simulink model "SdbTxSigProc"  (R2022b)
settings: Solver=FixedStepDiscrete  StartTime=0.0  StopTime=10.0  FixedStep=0.1  RelTol=1e-3
(part 3 of 4)

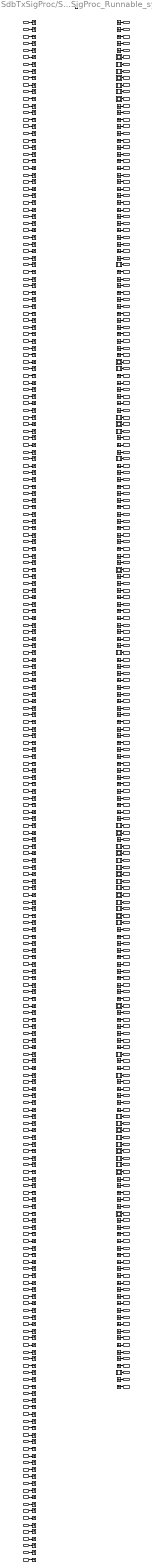
== system SdbTxSigProc/SdbTxSigProc_Runnable_sys ==
  AirTAftHvacDes_AirTAftHvacDes_read : Inport
  AirTFbFromClima_Ngcc_AirTFbFromClima_read : Inport  Port=2
  AmbTEstimd_Ngcc_AmbTEstimd_read : Inport  Port=3
  AmbTFildForClimaNgcc_AmbTFildForClima_read : Inport  Port=4
  AmbTFildForClimaQf_AmbTFildForClimaQf_read : Inport  Port=5
  CdsnProbltyToExt_CdsnProbltyToExt_read : Inport  Port=6
  ClimaActv_Ngcc_ClimaActv_read : Inport  Port=7
  ClimaActvAlrmSts_Ngcc_ClimaActvAlrmSts_read : Inport  Port=8
  ClimaCoolgPwrThermPred_ClimaCoolgPwrThermPred_read : Inport  Port=9
  ClimaDefrstMaxReq_ClimaDefrstMaxReq_read : Inport  Port=10
  ClimaOffReq_Ngcc_ClimaOffReq_read : Inport  Port=11
  ClimaPwrCns_Ngcc_ClimaPwrCns_read : Inport  Port=12
  ClimaPwrCnsPred_ClimaPwrCnsPred_read : Inport  Port=13
  ClimaPwrCritDes_Ngcc_ClimaPwrCritDes_read : Inport  Port=14
  ClimaPwrNormDes_Ngcc_ClimaPwrNormDes_read : Inport  Port=15
  ClimaStrtTi_ClimaStrtTi_read : Inport  Port=16
  ClimaSts_Ngcc_ClimaSts_read : Inport  Port=17
  ClimaTiOfEnd_Ngcc_ClimaTiOfEnd_read : Inport  Port=18
  ClimaTmrSts_ClimaTmrSts_read : Inport  Port=19
  ClimaWarn_ClimaWarn_read : Inport  Port=20
  ClngActv_ClngActv_read : Inport  Port=21
  CmptmtAirTEstimdAtRowFirstLoResl_Ngcc_CmptmtAirTEstimdAtRowFirstLoResl_read : Inport  Port=22
  CmptmtAirTEstimdExtd_Ngcc_CmptmtAirTEstimdExtd_read : Inport  Port=23
  CmptmtAirTFbExtd_CmptmtAirTFbExtd_read : Inport  Port=24
  CmptmtRelHumToExt_CmptmtRelHumToExt_read : Inport  Port=25
  CoolgStsForDisp_CoolgStsForDisp_read : Inport  Port=26
  CupHoldrActvAllwd_Ngcc_CupHoldrActvAllwd_read : Inport  Port=27
  CustClimaOvrdNotifForHmiCpt_CustClimaOvrdNotifForHmiCpt_read : Inport  Port=28
  DefrstReq_DefrstReq_read : Inport  Port=29
  DewVal_DewVal_read : Inport  Port=30
  DrvrSeatHeatgPwrAllwd_Ngcc_DrvrSeatHeatgPwrAllwd_read : Inport  Port=31
  DrvrSeatVentnPwrAllwd_Ngcc_DrvrSeatVentnPwrAllwd_read : Inport  Port=32
  EcoClimasts_Ngcc_EcoClimasts_read : Inport  Port=33
  EcoModForClimaActv_EcoModForClimaActv_read : Inport  Port=34
  EgyDesForClima_Ngcc_EgyDesForClima_read : Inport  Port=35
  EngRunngReqByClima_Ngcc_EngRunngReqByClima_read : Inport  Port=36
  EngSpdIncIdleForClima_Ngcc_EngSpdIncIdleForClima_read : Inport  Port=37
  EvaprTFrntFildForClima_EvaprTFrntFildForClima_read : Inport  Port=38
  EvaprTFrntFildQfForClima_EvaprTFrntFildQfForClima_read : Inport  Port=39
  HmiDefrstReqForClima_HmiDefrstReqForClima_read : Inport  Port=40
  HvacAirMFlowEstimd_Ngcc_HvacAirMFlowEstimd_read : Inport  Port=41
  HvacAirMFlowEstimdInHeatElm_HvacAirMFlowEstimdInHeatElm_read : Inport  Port=42
  HvacAirMFlowReEstimd_Ngcc_HvacAirMFlowReEstimd_read : Inport  Port=43
  HvacAirTFildForRowFirstAtFlrLe_HvacAirTFildForRowFirstAtFlrLe_read : Inport  Port=44
  HvacAirTFildForRowFirstAtFlrRi_HvacAirTFildForRowFirstAtFlrRi_read : Inport  Port=45
  HvacAirTFildForRowFirstAtVentLe_HvacAirTFildForRowFirstAtVentLe_read : Inport  Port=46
  HvacAirTFildForRowFirstAtVentRi_HvacAirTFildForRowFirstAtVentRi_read : Inport  Port=47
  HvacAirTFildForRowSecAtFlrLe_HvacAirTFildForRowSecAtFlrLe_read : Inport  Port=48
  HvacAirTFildForRowSecAtFlrRi_HvacAirTFildForRowSecAtFlrRi_read : Inport  Port=49
  HvacAirTFildForRowSecAtVentLe_HvacAirTFildForRowSecAtVentLe_read : Inport  Port=50
  HvacAirTFildForRowSecAtVentRi_HvacAirTFildForRowSecAtVentRi_read : Inport  Port=51
  HvacAirTForRowFirstAtFlrLeRaw_HvacAirTForRowFirstAtFlrLeRaw_read : Inport  Port=52
  HvacAirTForRowFirstAtFlrRiRaw_HvacAirTForRowFirstAtFlrRiRaw_read : Inport  Port=53
  HvacAirTForRowFirstAtVentRiRaw_HvacAirTForRowFirstAtVentRiRaw_read : Inport  Port=54
  HvacAirTForRowSecAtFlrLeRaw_HvacAirTForRowSecAtFlrLeRaw_read : Inport  Port=55
  HvacAirTForRowSecAtFlrRiRaw_HvacAirTForRowSecAtFlrRiRaw_read : Inport  Port=56
  HvacAirTForRowSecAtVentLeRaw_HvacAirTForRowSecAtVentLeRaw_read : Inport  Port=57
  HvacAirTForRowSecAtVentRiRaw_HvacAirTForRowSecAtVentRiRaw_read : Inport  Port=58
  HvacAirTReqAtRowFirstLe_HvacAirTReqAtRowFirstLe_read : Inport  Port=59
  HvacAirTReqAtRowFirstRi_HvacAirTReqAtRowFirstRi_read : Inport  Port=60
  HvacAirTReqAtRowSecLe_HvacAirTReqAtRowSecLe_read : Inport  Port=61
  HvacAirTReqAtRowSecRi_HvacAirTReqAtRowSecRi_read : Inport  Port=62
  HvacCondsorTspEvaprTFrnt_HvacCondsorTspEvaprTFrnt_read : Inport  Port=63
  HvacCoolgEnaFrnt_Ngcc_HvacCoolgEnaFrnt_read : Inport  Port=64
  HvacCoolgEnaRe_Ngcc_HvacCoolgEnaRe_read : Inport  Port=65
  HvacEvaprTSpFrnt_Ngcc_HvacEvaprTSpFrnt_read : Inport  Port=66
  HvacFlowFrntActForRowFirstAtVent_HvacFlowFrntActForRowFirstAtVent_read : Inport  Port=67
  HvacFlowFrntActForRowFirstAtVent_HvacFlowFrntActForRowFirstAtVentLe_read : Inport  Port=68
  HvacFlowFrntActForRowFirstAtVent_HvacFlowFrntActForRowFirstAtVentRi_read : Inport  Port=69
  HvacFlowFrntActForRowFirstAtVent_HvacFlowFrntActForRowFirstAtVentSideLe_read : Inport  Port=70
  HvacFlowFrntActForRowFirstAtVent_HvacFlowFrntActForRowFirstAtVentSideRi_read : Inport  Port=71
  HvacHeatgReqInt_HvacHeatgReqInt_read : Inport  Port=72
  HvacRecircAct_Ngcc_HvacRecircAct_read : Inport  Port=73
  HvEgyDesForClima_Ngcc_HvEgyDesForClima_read : Inport  Port=74
  InPm25SnsrActnReq_Ngcc_InPm25SnsrActnReq_read : Inport  Port=75
  IntPm25HiPopUp_Ngcc_IntPm25HiPopUp_read : Inport  Port=76
  LvlOfClimaCmft_Ngcc_LvlOfClimaCmft_read : Inport  Port=77
  PassSeatHeatgPwrAllwd_Ngcc_PassSeatHeatgPwrAllwd_read : Inport  Port=78
  PassSeatVentnPwrAllwd_Ngcc_PassSeatVentnPwrAllwd_read : Inport  Port=79
  PostDrvgClimaAvl_Ngcc_PostDrvgClimaAvl_read : Inport  Port=80
  PreClngActv_PreClngActv_read : Inport  Port=81
  PreClngNotif_PreClngNotif_read : Inport  Port=82
  PwrAllwdForElecAirVentn_Ngcc_PwrAllwdForElecAirVentn_read : Inport  Port=83
  PwrAllwdForSeatHeatg_PwrAllwdForSeatHeatg_read : Inport  Port=84
  PwrAllwdForSeatVent_PwrAllwdForSeatVent_read : Inport  Port=85
  PwrChrgDetdForPrkgHeatrElec_PwrChrgDetdForPrkgHeatrElec_read : Inport  Port=86
  PwrCnsHeatgElecPred_PwrCnsHeatgElecPred_read : Inport  Port=87
  PwrEstimdAtStbForClima_Ngcc_PwrEstimdAtStbForClima_read : Inport  Port=88
  RlyCmftForClimaReq_Ngcc_RlyCmftForClimaReq_read : Inport  Port=89
  RlyCrashForClimaReq_Ngcc_RlyCrashForClimaReq_read : Inport  Port=90
  SeatHeatgAutCdn_SeatHeatgAutCdn_read : Inport  Port=91
  SecRowLeSeatHeatgPwrAllwd_Ngcc_SecRowLeSeatHeatgPwrAllwd_read : Inport  Port=92
  SecRowLeSeatVentnPwrAllwd_Ngcc_SecRowLeSeatVentnPwrAllwd_read : Inport  Port=93
  SecRowRiSeatHeatgPwrAllwd_Ngcc_SecRowRiSeatHeatgPwrAllwd_read : Inport  Port=94
  SecRowRiSeatVentnPwrAllwd_Ngcc_SecRowRiSeatVentnPwrAllwd_read : Inport  Port=95
  SteerWhlHeatgAllwd_SteerWhlHeatgAllwd_read : Inport  Port=96
  SteerWhlHeatgDurgClima_Ngcc_SteerWhlHeatgDurgClima_read : Inport  Port=97
  UBoostReqByClima_Ngcc_UBoostReqByClima_read : Inport  Port=98
  VehMktForPmSnsr_Ngcc_VehMktForPmSnsr_read : Inport  Port=99
  WindDewUppr_WindDewTUppr_read : Inport  Port=100
  WindIceDetdFrnt_Ngcc_WindIceDetdFrnt_read : Inport  Port=101
  WindIceDetdRe_Ngcc_WindIceDetdRe_read : Inport  Port=102
  WindT_WindT_read : Inport  Port=103
  HvacAirTForRowFirstAtVentLeRaw_HvacAirTForRowFirstAtVentLeRaw_read : Inport  Port=104
  ClimaStStrtUp_ClimaStStrtUp_read : Inport  Port=105
  AACAirClngActnNgcc_AACAirClngActnNgcc_read : Inport  Port=106
  AirSrcReqNgcc_AirSrcReqNgcc_read : Inport  Port=107
  AirTAftHvacDesFirstLe_AirTAftHvacDesFirstLe_read : Inport  Port=108
  AirTAftHvacDesFirstRi_AirTAftHvacDesFirstRi_read : Inport  Port=109
  AirTAftHvacDesRe_AirTAftHvacDesRe_read : Inport  Port=110
  AirTAftHvacDesSecLe_AirTAftHvacDesSecLe_read : Inport  Port=111
  AirTFromHeatgEstimdFirstLeForCCM_AirTFromHeatgEstimdFirstLeForCCM_read : Inport  Port=112
  AirTFromHeatgEstimdFirstRiForCCM_AirTFromHeatgEstimdFirstRiForCCM_read : Inport  Port=113
  AirTFromHeatgEstimdForCCM_AirTFromHeatgEstimd_read : Inport  Port=114
  AirTFromHeatgEstimdReForCCM_AirTFromHeatgEstimdReForCCM_read : Inport  Port=115
  AirTFromHeatgEstimdSecLeForCCM_AirTFromHeatgEstimdSecLeForCCM_read : Inport  Port=116
  AutOxygenSplyStsFrmClimaNgcc_AutOxygenSplyStsFrmClimaNgcc_read : Inport  Port=117
  CcmAACAirClngStsNgcc_CcmAACAirClngStsNgcc_read : Inport  Port=118
  CCMDataTraceZCUP1Ngcc_CCMDataTraceZCUP1Ngcc_read : Inport  Port=119
  CCMDataTraceZCUP2Ngcc_CCMDataTraceZCUP2Ngcc_read : Inport  Port=120
  CCMDataTraceZCUP3Ngcc_CCMDataTraceZCUP3Ngcc_read : Inport  Port=121
  CCMDataTraceZCUP4Ngcc_CCMDataTraceZCUP4Ngcc_read : Inport  Port=122
  CCMDataTraceZCUP5Ngcc_CCMDataTraceZCUP5Ngcc_read : Inport  Port=123
  CCMDataTraceZCUP6Ngcc_CCMDataTraceZCUP6Ngcc_read : Inport  Port=124
  CCMDataTraceZCUP7Ngcc_CCMDataTraceZCUP7Ngcc_read : Inport  Port=125
  CcmElecAirDirCrtlReqSecCnsLePosXNgcc_CcmElecAirDirCrtlReqSecCnsLePosXNgcc_read : Inport  Port=126
  CcmElecAirDirCrtlReqSecCnsLePosYNgcc_CcmElecAirDirCrtlReqSecCnsLePosYNgcc_read : Inport  Port=127
  CcmElecAirDirCrtlReqSecCnsRiPosXNgcc_CcmElecAirDirCrtlReqSecCnsRiPosXNgcc_read : Inport  Port=128
  CcmElecAirDirCrtlReqSecCnsRiPosYNgcc_CcmElecAirDirCrtlReqSecCnsRiPosYNgcc_read : Inport  Port=129
  CCMFragCh1OvrdDyNgcc_CCMFragCh1OvrdDyNgcc_read : Inport  Port=130
  CCMFragCh2OvrdDyNgcc_CCMFragCh2OvrdDyNgcc_read : Inport  Port=131
  CCMFragCh3OvrdDyNgcc_CCMFragCh3OvrdDyNgcc_read : Inport  Port=132
  CCMFragCh4OvrdDyNgcc_CCMFragCh4OvrdDyNgcc_read : Inport  Port=133
  CCMFragCh5OvrdDyNgcc_CCMFragCh5OvrdDyNgcc_read : Inport  Port=134
  CCMFragraCh1AvlTiPrecNgcc_CCMFragraCh1AvlTiPrecNgcc_read : Inport  Port=135
  CCMFragraCh1AvlTiPrecNgcc_CCMFragraCh1AvlTiPrecNgcc_IsUpdated_read : Inport  Port=136
  CCMFragraCh2AvlTiPrecNgcc_CCMFragraCh2AvlTiPrecNgcc_read : Inport  Port=137
  CCMFragraCh2AvlTiPrecNgcc_CCMFragraCh2AvlTiPrecNgcc_IsUpdated_read : Inport  Port=138
  CCMFragraCh3AvlTiPrecNgcc_CCMFragraCh3AvlTiPrecNgcc_read : Inport  Port=139
  CCMFragraCh3AvlTiPrecNgcc_CCMFragraCh3AvlTiPrecNgcc_IsUpdated_read : Inport  Port=140
  CCMFragraCh4AvlTiPrecNgcc_CCMFragraCh4AvlTiPrecNgcc_read : Inport  Port=141
  CCMFragraCh4AvlTiPrecNgcc_CCMFragraCh4AvlTiPrecNgcc_IsUpdated_read : Inport  Port=142
  CCMFragraCh5AvlTiPrecNgcc_CCMFragraCh5AvlTiPrecNgcc_read : Inport  Port=143
  CCMFragraCh5AvlTiPrecNgcc_CCMFragraCh5AvlTiPrecNgcc_IsUpdated_read : Inport  Port=144
  CcmWbmMasterReqLeLin1Ngcc_CcmWbmMasterReqLeLin1Ngcc_read : Inport  Port=145
  CcmWbmMasterReqRiLin1Ngcc_CcmWbmMasterReqRiLin1Ngcc_read : Inport  Port=146
  CdsnProblty_CdsnProblty_read : Inport  Port=147
  ChgFilElmRstNgcc_ChgFilElmRstNgcc_read : Inport  Port=148
  ClimaAtRowSecActvNgcc_ClimaAtRowSecActv_read : Inport  Port=149
  ClimaAtRowSecActvNgcc_ClimaAtRowSecActvLe_read : Inport  Port=150
  ClimaAtRowSecActvNgcc_ClimaAtRowSecActvRi_read : Inport  Port=151
  ClimaCtrlActvNgcc_ClimaCtrlActv_read : Inport  Port=152
  ClimaManModCmftPopUpReqNgcc_ClimaManModCmftPopUpReqNgcc_read : Inport  Port=153
  CmptmtAirDistbnRspNgcc_CmptmtAirDistbnRspNgcc_read : Inport  Port=154
  CmptmtAirDistbnRspNgcc_CmptmtAirDistbnRspNgcc_IsUpdated_read : Inport  Port=155
  CmptmtOxygenConcLvlFrmClimaNgcc_CmptmtOxygenConcLvlFrmClimaNgcc_read : Inport  Port=156
  CmptmtOxygenConcVluFrmClimaNgcc_CmptmtOxygenConcVluFrmClimaNgcc_read : Inport  Port=157
  CoupldCtrlErrAtRowFirstLeNgcc_CoupldCtrlErrAtRowFirstLe_read : Inport  Port=158
  CoupldCtrlErrAtRowFirstRiNgcc_CoupldCtrlErrAtRowFirstRi_read : Inport  Port=159
  CoupldCtrlErrAtRowSecLeNgcc_CoupldCtrlErrAtRowSecLe_read : Inport  Port=160
  CoupldCtrlErrAtRowSecRiNgcc_CoupldCtrlErrAtRowSecRi_read : Inport  Port=161
  DutyCmdForMosBlw_DutyCmdForMosBlw_read : Inport  Port=162
  DutyCmdForPWMBlw_DutyCmdForPWMBlw_read : Inport  Port=163
  ExtVentToastFrmClima_Ngcc_ExtVentToastFrmClima_read : Inport  Port=164
  FrntClimaManModCmftStsNgcc_FrntClimaManModCmftStsNgcc_read : Inport  Port=165
  HighTempCleanRunningStsNgcc_HighTempCleanRunningStsNgcc_read : Inport  Port=166
  HighTempCleanSettingStsNgcc_HighTempCleanSettingStsNgcc_read : Inport  Port=167
  HvacAirTReAtFlrLeRaw_HvacAirTReAtFlrLeRaw_read : Inport  Port=168
  HvacAirTReAtVentLeRaw_HvacAirTReAtVentLeRaw_read : Inport  Port=169
  HvacEvaprTSpReNgcc_HvacEvaprTSpReNgcc_read : Inport  Port=170
  HvacFanLvlFbNgcc_HvacFanLvlFbNgcc_read : Inport  Port=171
  HvacFanLvlFbReNgcc_HvacFanLvlFbReNgcc_read : Inport  Port=172
  HvacHeatElmAirMFlowEstimdFirstLeNgcc_HvacHeatElmAirMFlowEstimdFirstLeNgcc_read : Inport  Port=173
  HvacHeatElmAirMFlowEstimdFirstRiNgcc_HvacHeatElmAirMFlowEstimdFirstRiNgcc_read : Inport  Port=174
  HvacHeatElmAirMFlowEstimdSecLeNgcc_HvacHeatElmAirMFlowEstimdSecLeNgcc_read : Inport  Port=175
  HvacHeatgReqReInt_HvacHeatgReqReInt_read : Inport  Port=176
  HvacHexAirTForRowFirstLeRaw_HvacHexAirTForRowFirstLeRaw_read : Inport  Port=177
  HvacHexAirTForRowFirstRiRaw_HvacHexAirTForRowFirstRiRaw_read : Inport  Port=178
  HvacHexAirTForRowSecLeRaw_HvacHexAirTForRowSecLeRaw_read : Inport  Port=179
  KeySynchrnztnLclNgcc_KeySynchrnztnLclNgcc_read : Inport  Port=180
  OutlSovActvStsNgcc_OutlSovActvStsNgcc_read : Inport  Port=181
  OxygenConcSnsrActnReqNgcc_OxygenConcSnsrActnReqNgcc_read : Inport  Port=182
  OxygenConcSnsrStsFrmClimaNgcc_OxygenConcSnsrStsFrmClimaNgcc_read : Inport  Port=183
  OxygenSplyAvlStsFrmClimaNgcc_OxygenSplyAvlStsFrmClimaNgcc_read : Inport  Port=184
  OxygenSplyErrFrmClimaNgcc_OxygenSplyErrFrmClimaNgcc_read : Inport  Port=185
  OxygenSplyPopUpReqNgcc_OxygenSplyPopUpReqNgcc_read : Inport  Port=186
  OxygenSplyPwrAllwdNgcc_OxygenSplyPwrAllwdNgcc_read : Inport  Port=187
  OxygenSplyReqFaildNgcc_OxygenSplyReqFaildNgcc_read : Inport  Port=188
  OxygenSplyReqNgcc_OxygenSplyReqNgcc_read : Inport  Port=189
  OxygenSplyVoiceFbNgcc_OxygenSplyVoiceFbNgcc_read : Inport  Port=190
  PrkgClimaEngStReqNgcc_PrkgClimaEngStReqNgcc_read : Inport  Port=191
  PwrCnsForHvacFanFrntNgcc_PwrCnsForHvacFanFrnt_read : Inport  Port=192
  PwrCnsForHvacFanReNgcc_PwrCnsForHvacFanRe_read : Inport  Port=193
  ReClimaManModCmftStsNgcc_ReClimaManModCmftStsNgcc_read : Inport  Port=194
  RemOxygenSplyActvFaildNgcc_RemOxygenSplyActvFaildNgcc_read : Inport  Port=195
  TCSCmprStrtFltNgcc_TCSCmprStrtFltNgcc_read : Inport  Port=196
  TCScoolgGrdReqNgcc_TCScoolgGrdReqNgcc_read : Inport  Port=197
  TCSCooltFlowReq1Ngcc_TCSCooltFlowReq1Ngcc_read : Inport  Port=198
  TCSCooltTReq1Ngcc_TCSCooltTReq1Ngcc_read : Inport  Port=199
  TCSDoorStsNgcc_TCSDoorStsNgcc_read : Inport  Port=200
  TCSDoorTopSts1Ngcc_TCSDoorTopSts1Ngcc_read : Inport  Port=201
  TcsEcoModSts1Ngcc_TcsEcoModSts1Ngcc_read : Inport  Port=202
  TcsFltNewSts1Ngcc_TcsFltNewSts1Ngcc_read : Inport  Port=203
  TCSFltStsNgcc_TCSFltStsNgcc_read : Inport  Port=204
  TCSModSwtStsNgcc_TCSModSwtStsNgcc_read : Inport  Port=205
  TCSnsrTStsNgcc_TCSnsrTStsNgcc_read : Inport  Port=206
  TCSRadrTreqNgcc_TCSRadrTreqNgcc_read : Inport  Port=207
  TCSRadrTStsNgcc_TCSRadrTStsNgcc_read : Inport  Port=208
  TCSTmrOverStsNgcc_TCSTmrOverStsNgcc_read : Inport  Port=209
  TCSTmrStrtStsNgcc_TCSTmrStrtStsNgcc_read : Inport  Port=210
  TCSTNowStsNgcc_TCSTNowStsNgcc_read : Inport  Port=211
  TgtHvacAirTForMaxTZoneNgcc_TgtHvacAirTForMaxTZoneNgcc_read : Inport  Port=212
  TgtHvacAirTForRowFirstLeNgcc_TgtHvacAirTForRowFirstLeNgcc_read : Inport  Port=213
  TgtHvacAirTForRowFirstRiNgcc_TgtHvacAirTForRowFirstRiNgcc_read : Inport  Port=214
  TgtHvacAirTForRowSecLeNgcc_TgtHvacAirTForRowSecLeNgcc_read : Inport  Port=215
  TgtHvacAirTForRowSecRiNgcc_TgtHvacAirTForRowSecRiNgcc_read : Inport  Port=216
  ThermoTSteDeoSts1Ngcc_ThermoTSteDeoSts1Ngcc_read : Inport  Port=217
  ThrdRowLeSeatHeatgPwrAllwdNgcc_ThrdRowLeSeatHeatgPwrAllwdNgcc_read : Inport  Port=218
  ThrdRowLeSeatVentnPwrAllwdNgcc_ThrdRowLeSeatVentnPwrAllwdNgcc_read : Inport  Port=219
  ThrdRowRiSeatHeatgPwrAllwdNgcc_ThrdRowRiSeatHeatgPwrAllwdNgcc_read : Inport  Port=220
  ThrdRowRiSeatVentnPwrAllwdNgcc_ThrdRowRiSeatVentnPwrAllwdNgcc_read : Inport  Port=221
  TiOfDeactvnForClimaCtrlNgcc_TiOfDeactvnForClimaCtrl_read : Inport  Port=222
  VMMChngNdMtnNgcc_VMMChngNdMtnNgcc_read : Inport  Port=223
  function : TriggerPort  TriggerType=function-call  VariantControl=(inherit)  SampleTimeType=periodic  SampleTime=-1
  Ground_{1..5,13..35,37..49,52..57,61..63,65..79,81..91,93..116,119,132..142,144..149,151..152,154..158,168..172,174..195,197..198} (154 alike) : Ground
  Ground_10 : Subsystem
  Ground_11 : Subsystem
  Ground_117 : Subsystem
  Ground_118 : Subsystem
  Ground_12 : Subsystem
  Ground_120 : Subsystem
  Ground_121 : Subsystem
  Ground_122 : Subsystem
  Ground_123 : Subsystem
  Ground_124 : Subsystem
  Ground_125 : Subsystem
  Ground_126 : Subsystem
  Ground_127 : Subsystem
  Ground_128 : Subsystem
  Ground_129 : Subsystem
  Ground_130 : Subsystem
  Ground_131 : Subsystem
  Ground_143 : Subsystem
  Ground_150 : Subsystem
  Ground_153 : Subsystem
  Ground_159 : Subsystem
  Ground_160 : Subsystem
  Ground_161 : Subsystem
  Ground_162 : Subsystem
  Ground_163 : Subsystem
  Ground_164 : Subsystem
  Ground_165 : Subsystem
  Ground_166 : Subsystem
  Ground_167 : Subsystem
  Ground_173 : Subsystem
  Ground_196 : Subsystem
  Ground_36 : Subsystem
  Ground_50 : Subsystem
  Ground_51 : Subsystem
  Ground_58 : Subsystem
  Ground_59 : Subsystem
  Ground_6 : Subsystem
  Ground_60 : Subsystem
  Ground_64 : Subsystem
  Ground_7 : Subsystem
  Ground_8 : Subsystem
  Ground_80 : Subsystem
  Ground_9 : Subsystem
  Ground_92 : Subsystem
  Terminator_{1..223} (223 alike) : Terminator
  AACAirClngActn_AACAirClngActn_write : Outport
      settings: EnsureOutportIsVirtual=on
  AirSrcReq_AirSrcReq_write : Outport  Port=2
      settings: EnsureOutportIsVirtual=on
  AmbTFildForClima_AmbTFildForClima_write : Outport  Port=3
      settings: EnsureOutportIsVirtual=on
  AutOxygenSplyStsFrmClima_AutOxygenSplyStsFrmClima_write : Outport  Port=4
      settings: EnsureOutportIsVirtual=on
  CcmAACAirClngSts_CcmAACAirClngSts_write : Outport  Port=5
      settings: EnsureOutportIsVirtual=on
  CCMDataTraceZCUP1_CCMDataTraceZCUP1_write : Outport  Port=6
      settings: EnsureOutportIsVirtual=on
  CCMDataTraceZCUP2_CCMDataTraceZCUP2_write : Outport  Port=7
      settings: EnsureOutportIsVirtual=on
  CCMDataTraceZCUP3_CCMDataTraceZCUP3_write : Outport  Port=8
      settings: EnsureOutportIsVirtual=on
  CCMDataTraceZCUP4_CCMDataTraceZCUP4_write : Outport  Port=9
      settings: EnsureOutportIsVirtual=on
  CCMDataTraceZCUP5_CCMDataTraceZCUP5_write : Outport  Port=10
      settings: EnsureOutportIsVirtual=on
  CCMDataTraceZCUP6_CCMDataTraceZCUP6_write : Outport  Port=11
      settings: EnsureOutportIsVirtual=on
  CCMDataTraceZCUP7_CCMDataTraceZCUP7_write : Outport  Port=12
      settings: EnsureOutportIsVirtual=on
  CcmElecAirDirCrtlReqSecCnsLePosX_CcmElecAirDirCrtlReqSecCnsLePosX_write : Outport  Port=13
      settings: EnsureOutportIsVirtual=on
  CcmElecAirDirCrtlReqSecCnsLePosY_CcmElecAirDirCrtlReqSecCnsLePosY_write : Outport  Port=14
      settings: EnsureOutportIsVirtual=on
  CcmElecAirDirCrtlReqSecCnsRiPosX_CcmElecAirDirCrtlReqSecCnsRiPosX_write : Outport  Port=15
      settings: EnsureOutportIsVirtual=on
  CcmElecAirDirCrtlReqSecCnsRiPosY_CcmElecAirDirCrtlReqSecCnsRiPosY_write : Outport  Port=16
      settings: EnsureOutportIsVirtual=on
  CCMFragCh1OvrdDy_CCMFragCh1OvrdDy_write : Outport  Port=17
      settings: EnsureOutportIsVirtual=on
  CCMFragCh2OvrdDy_CCMFragCh2OvrdDy_write : Outport  Port=18
      settings: EnsureOutportIsVirtual=on
  CCMFragCh3OvrdDy_CCMFragCh3OvrdDy_write : Outport  Port=19
      settings: EnsureOutportIsVirtual=on
  CCMFragCh4OvrdDy_CCMFragCh4OvrdDy_write : Outport  Port=20
      settings: EnsureOutportIsVirtual=on
  CCMFragCh5OvrdDy_CCMFragCh5OvrdDy_write : Outport  Port=21
      settings: EnsureOutportIsVirtual=on
  CCMFragraCh1AvlTiPrec_CCMFragraCh1AvlTiPrec_write : Outport  Port=22
      settings: EnsureOutportIsVirtual=on
  CCMFragraCh2AvlTiPrec_CCMFragraCh2AvlTiPrec_write : Outport  Port=23
      settings: EnsureOutportIsVirtual=on
  CCMFragraCh3AvlTiPrec_CCMFragraCh3AvlTiPrec_write : Outport  Port=24
      settings: EnsureOutportIsVirtual=on
  CCMFragraCh4AvlTiPrec_CCMFragraCh4AvlTiPrec_write : Outport  Port=25
      settings: EnsureOutportIsVirtual=on
  CCMFragraCh5AvlTiPrec_CCMFragraCh5AvlTiPrec_write : Outport  Port=26
      settings: EnsureOutportIsVirtual=on
  CcmWbmMasterReqLeLin1_CcmWbmMasterReqLeLin1_write : Outport  Port=27
      settings: EnsureOutportIsVirtual=on
  CcmWbmMasterReqRiLin1_CcmWbmMasterReqRiLin1_write : Outport  Port=28
      settings: EnsureOutportIsVirtual=on
  ChgFilElmRst_ChgFilElmRst_write : Outport  Port=29
      settings: EnsureOutportIsVirtual=on
  ClimaAtRowSecActv_ClimaAtRowSecActv_write : Outport  Port=30
      settings: EnsureOutportIsVirtual=on
  ClimaCtrlActv_ClimaCtrlActv_write : Outport  Port=31
      settings: EnsureOutportIsVirtual=on
  ClimaCtrlTiOfDeactvn_ClimaCtrlTiOfDeactvn_write : Outport  Port=32
      settings: EnsureOutportIsVirtual=on
  ClimaEstimdTForHeat_ClimaEstimdTForHeat_write : Outport  Port=33
      settings: EnsureOutportIsVirtual=on
  ClimaEstimdTForReHeat_ClimaEstimdTForReHeat_write : Outport  Port=34
      settings: EnsureOutportIsVirtual=on
  ClimaManModCmftPopUpReq_ClimaManModCmftPopUpReq_write : Outport  Port=35
      settings: EnsureOutportIsVirtual=on
  CmptmtAirDistbnRsp_CmptmtAirDistbnRsp_write : Outport  Port=36
      settings: EnsureOutportIsVirtual=on
  CmptmtOxygenConcLvlFrmClima_CmptmtOxygenConcLvlFrmClima_write : Outport  Port=37
      settings: EnsureOutportIsVirtual=on
  CmptmtOxygenConcVluFrmClima_CmptmtOxygenConcVluFrmClima_write : Outport  Port=38
      settings: EnsureOutportIsVirtual=on
  CoupldCtrlErrAtRowFirstLe_CoupldCtrlErrAtRowFirstLe_write : Outport  Port=39
      settings: EnsureOutportIsVirtual=on
  CoupldCtrlErrAtRowFirstRi_CoupldCtrlErrAtRowFirstRi_write : Outport  Port=40
      settings: EnsureOutportIsVirtual=on
  CoupldCtrlErrAtRowSecLe_CoupldCtrlErrAtRowSecLe_write : Outport  Port=41
      settings: EnsureOutportIsVirtual=on
  CoupldCtrlErrAtRowSecRi_CoupldCtrlErrAtRowSecRi_write : Outport  Port=42
      settings: EnsureOutportIsVirtual=on
  FrntBlwSpdReq_FrntBlwSpdReq_write : Outport  Port=43
      settings: EnsureOutportIsVirtual=on
  FrntClimaManModCmftSts_FrntClimaManModCmftSts_write : Outport  Port=44
      settings: EnsureOutportIsVirtual=on
  HeatrAirTReqFirstLe_HeatrAirTReqFirstLe_write : Outport  Port=45
      settings: EnsureOutportIsVirtual=on
  HeatrAirTReqFirstRi_HeatrAirTReqFirstRi_write : Outport  Port=46
      settings: EnsureOutportIsVirtual=on
  HeatrAirTReqSecLe_HeatrAirTReqSecLe_write : Outport  Port=47
      settings: EnsureOutportIsVirtual=on
  HighTempCleanRunningSts_HighTempCleanRunningSts_write : Outport  Port=48
      settings: EnsureOutportIsVirtual=on
  HighTempCleanSettingSts_HighTempCleanSettingSts_write : Outport  Port=49
      settings: EnsureOutportIsVirtual=on
  HvacAirTForRowThrdAtFlrRi_HvacAirTForRowThrdAtFlrRi_write : Outport  Port=50
      settings: EnsureOutportIsVirtual=on
  HvacAirTForRowThrdAtVentRi_HvacAirTForRowThrdAtVentRi_write : Outport  Port=51
      settings: EnsureOutportIsVirtual=on
  HvacEvaprTSpRe_HvacEvaprTSpRe_write : Outport  Port=52
      settings: EnsureOutportIsVirtual=on
  HvacFanLvlFbFrnt_HvacFanLvlFbFrnt_write : Outport  Port=53
      settings: EnsureOutportIsVirtual=on
  HvacFanLvlFbRe_HvacFanLvlFbRe_write : Outport  Port=54
      settings: EnsureOutportIsVirtual=on
  HvacHeatElmAirMFlowEstimdFirstLe_HvacHeatElmAirMFlowEstimdFirstLe_write : Outport  Port=55
      settings: EnsureOutportIsVirtual=on
  HvacHeatElmAirMFlowEstimdFirstRi_HvacHeatElmAirMFlowEstimdFirstRi_write : Outport  Port=56
      settings: EnsureOutportIsVirtual=on
  HvacHeatElmAirMFlowEstimdSecLe_HvacHeatElmAirMFlowEstimdSecLe_write : Outport  Port=57
      settings: EnsureOutportIsVirtual=on
  HvacHexAirTFirstLe_HvacHexAirTFirstLe_write : Outport  Port=58
      settings: EnsureOutportIsVirtual=on
  HvacHexAirTFirstRi_HvacHexAirTFirstRi_write : Outport  Port=59
      settings: EnsureOutportIsVirtual=on
  HvacHexAirTSecLe_HvacHexAirTSecLe_write : Outport  Port=60
      settings: EnsureOutportIsVirtual=on
  HvahAirTsp_HvahAirTsp_write : Outport  Port=61
      settings: EnsureOutportIsVirtual=on
  HvahHeatgReq_HvahHeatgReq_write : Outport  Port=62
      settings: EnsureOutportIsVirtual=on
  HwFrntBlwPwmReq_HwFrntBlwPwmReq_write : Outport  Port=63
      settings: EnsureOutportIsVirtual=on
  KeySynchrnztnLcl_KeySynchrnztnLcl_write : Outport  Port=64
      settings: EnsureOutportIsVirtual=on
  MistRiskLvl_MistRiskLvl_write : Outport  Port=65
      settings: EnsureOutportIsVirtual=on
  OutlSovActvSts_OutlSovActvSts_write : Outport  Port=66
      settings: EnsureOutportIsVirtual=on
  OxygenConcSnsrActnReq_OxygenConcSnsrActnReq_write : Outport  Port=67
      settings: EnsureOutportIsVirtual=on
  OxygenConcSnsrStsFrmClima_OxygenConcSnsrStsFrmClima_write : Outport  Port=68
      settings: EnsureOutportIsVirtual=on
  OxygenSplyAvlStsFrmClima_OxygenSplyAvlStsFrmClima_write : Outport  Port=69
      settings: EnsureOutportIsVirtual=on
  OxygenSplyErrFrmClima_OxygenSplyErrFrmClima_write : Outport  Port=70
      settings: EnsureOutportIsVirtual=on
  OxygenSplyPopUpReq_OxygenSplyPopUpReq_write : Outport  Port=71
      settings: EnsureOutportIsVirtual=on
  OxygenSplyPwrAllwd_OxygenSplyPwrAllwd_write : Outport  Port=72
      settings: EnsureOutportIsVirtual=on
  OxygenSplyReq_OxygenSplyReq_write : Outport  Port=73
      settings: EnsureOutportIsVirtual=on
  OxygenSplyReqFaild_OxygenSplyReqFaild_write : Outport  Port=74
      settings: EnsureOutportIsVirtual=on
  OxygenSplyVoiceFb_OxygenSplyVoiceFb_write : Outport  Port=75
      settings: EnsureOutportIsVirtual=on
  PrkgClimaEngStReq_PrkgClimaEngStReq_write : Outport  Port=76
      settings: EnsureOutportIsVirtual=on
  PwrCnsForHvacFanFrnt_PwrCnsForHvacFanFrnt_write : Outport  Port=77
      settings: EnsureOutportIsVirtual=on
  PwrCnsForHvacFanRe_PwrCnsForHvacFanRe_write : Outport  Port=78
      settings: EnsureOutportIsVirtual=on
  ReClimaManModCmftSts_ReClimaManModCmftSts_write : Outport  Port=79
      settings: EnsureOutportIsVirtual=on
  RemClimaElecDefrstReq_RemClimaElecDefrstReq_write : Outport  Port=80
      settings: EnsureOutportIsVirtual=on
  RemOxygenSplyActvFaild_RemOxygenSplyActvFaild_write : Outport  Port=81
      settings: EnsureOutportIsVirtual=on
  TCSCmprStrtFlt_TCSCmprStrtFlt_write : Outport  Port=82
      settings: EnsureOutportIsVirtual=on
  TCSCmprStrtFlt1_TCSCmprStrtFlt1_write : Outport  Port=83
      settings: EnsureOutportIsVirtual=on
  TCScoolgGrdReq_TCScoolgGrdReq_write : Outport  Port=84
      settings: EnsureOutportIsVirtual=on
  TCScoolgGrdReq1_TCScoolgGrdReq1_write : Outport  Port=85
      settings: EnsureOutportIsVirtual=on
  TCSCooltFlowReq1_TCSCooltFlowReq1_write : Outport  Port=86
      settings: EnsureOutportIsVirtual=on
  TCSCooltTReq1_TCSCooltTReq1_write : Outport  Port=87
      settings: EnsureOutportIsVirtual=on
  TCSDoorSts_TCSDoorSts_write : Outport  Port=88
      settings: EnsureOutportIsVirtual=on
  TCSDoorSts1_TCSDoorSts1_write : Outport  Port=89
      settings: EnsureOutportIsVirtual=on
  TCSDoorTopSts1_TCSDoorTopSts1_write : Outport  Port=90
      settings: EnsureOutportIsVirtual=on
  TcsEcoModSts1_Element_write : Outport  Port=91
      settings: EnsureOutportIsVirtual=on
  TcsFltNewSts1_TcsFltNewSts1_write : Outport  Port=92
      settings: EnsureOutportIsVirtual=on
  TCSFltSts_TCSFltSts_write : Outport  Port=93
      settings: EnsureOutportIsVirtual=on
  TCSFltSts1_TCSFltSts1_write : Outport  Port=94
      settings: EnsureOutportIsVirtual=on
  TCSModSwtSts_TCSModSwtSts_write : Outport  Port=95
      settings: EnsureOutportIsVirtual=on
  TCSModSwtSts1_TCSModSwtSts1_write : Outport  Port=96
      settings: EnsureOutportIsVirtual=on
  TCSnsrTSts_TCSnsrTSts_write : Outport  Port=97
      settings: EnsureOutportIsVirtual=on
  TCSnsrTSts1_TCSnsrTSts1_write : Outport  Port=98
      settings: EnsureOutportIsVirtual=on
  TCSRadrTreq_TCSRadrTreq_write : Outport  Port=99
      settings: EnsureOutportIsVirtual=on
  TCSRadrTreq1_TCSRadrTreq1_write : Outport  Port=100
      settings: EnsureOutportIsVirtual=on
  TCSRadrTSts_TCSRadrTSts_write : Outport  Port=101
      settings: EnsureOutportIsVirtual=on
  TCSRadrTSts1_TCSRadrTSts1_write : Outport  Port=102
      settings: EnsureOutportIsVirtual=on
  TCSTmrOverSts_TCSTmrOverSts_write : Outport  Port=103
      settings: EnsureOutportIsVirtual=on
  TCSTmrOverSts1_TCSTmrOverSts1_write : Outport  Port=104
      settings: EnsureOutportIsVirtual=on
  TCSTmrStrtSts_TCSTmrStrtSts_write : Outport  Port=105
      settings: EnsureOutportIsVirtual=on
  TCSTmrStrtSts1_TCSTmrStrtSts1_write : Outport  Port=106
      settings: EnsureOutportIsVirtual=on
  TCSTNowSts_TCSTNowSts_write : Outport  Port=107
      settings: EnsureOutportIsVirtual=on
  TCSTNowSts1_TCSTNowSts1_write : Outport  Port=108
      settings: EnsureOutportIsVirtual=on
  TgtHvacAirTForMaxTZone_TgtHvacAirTForMaxTZone_write : Outport  Port=109
      settings: EnsureOutportIsVirtual=on
  ThermoTSteDeoSts1_ThermoTSteDeoSts1_write : Outport  Port=110
      settings: EnsureOutportIsVirtual=on
  ThrdRowLeSeatHeatgPwrAllwd_ThrdRowLeSeatHeatgPwrAllwd_write : Outport  Port=111
      settings: EnsureOutportIsVirtual=on
  ThrdRowLeSeatVentnPwrAllwd_ThrdRowLeSeatVentnPwrAllwd_write : Outport  Port=112
      settings: EnsureOutportIsVirtual=on
  ThrdRowRiSeatHeatgPwrAllwd_ThrdRowRiSeatHeatgPwrAllwd_write : Outport  Port=113
      settings: EnsureOutportIsVirtual=on
  ThrdRowRiSeatVentnPwrAllwd_ThrdRowRiSeatVentnPwrAllwd_write : Outport  Port=114
      settings: EnsureOutportIsVirtual=on
  TiOfDeactvnForClimaCtrl_TiOfDeactvnForClimaCtrl_write : Outport  Port=115
      settings: EnsureOutportIsVirtual=on
  VMMChngNdMtn_VMMChngNdMtn_write : Outport  Port=116
      settings: EnsureOutportIsVirtual=on
  AirTFbFromClima_AirTFbFromClima_write : Outport  Port=117
      settings: EnsureOutportIsVirtual=on
  AmbTEstimd_AmbTEstimd_write : Outport  Port=118
      settings: EnsureOutportIsVirtual=on
  CarTiIntForClima_CarTiIntForClima_write : Outport  Port=119
      settings: EnsureOutportIsVirtual=on
  CcmCaliSig1_CcmCaliSig1_write : Outport  Port=120
      settings: EnsureOutportIsVirtual=on
  CcmCaliSig10_CcmCaliSig10_write : Outport  Port=121
      settings: EnsureOutportIsVirtual=on
  CcmCaliSig11_CcmCaliSig11_write : Outport  Port=122
      settings: EnsureOutportIsVirtual=on
  CcmCaliSig12_CcmCaliSig12_write : Outport  Port=123
      settings: EnsureOutportIsVirtual=on
  CcmCaliSig2_CcmCaliSig2_write : Outport  Port=124
      settings: EnsureOutportIsVirtual=on
  CcmCaliSig3_CcmCaliSig3_write : Outport  Port=125
      settings: EnsureOutportIsVirtual=on
  CcmCaliSig4_CcmCaliSig4_write : Outport  Port=126
      settings: EnsureOutportIsVirtual=on
  CcmCaliSig5_CcmCaliSig5_write : Outport  Port=127
      settings: EnsureOutportIsVirtual=on
  CcmCaliSig6_CcmCaliSig6_write : Outport  Port=128
      settings: EnsureOutportIsVirtual=on
  CcmCaliSig7_CcmCaliSig7_write : Outport  Port=129
      settings: EnsureOutportIsVirtual=on
  CcmCaliSig8_CcmCaliSig8_write : Outport  Port=130
      settings: EnsureOutportIsVirtual=on
  CcmCaliSig9_CcmCaliSig9_write : Outport  Port=131
      settings: EnsureOutportIsVirtual=on
  ClimaActv_ClimaActv_write : Outport  Port=132
      settings: EnsureOutportIsVirtual=on
  ClimaActvAlrmSts_ClimaActvAlrmSts_write : Outport  Port=133
      settings: EnsureOutportIsVirtual=on
  ClimaCmptSts_ClimaCmptSts_write : Outport  Port=134
      settings: EnsureOutportIsVirtual=on
  ClimaDefrstSts_ClimaDefrstSts_write : Outport  Port=135
      settings: EnsureOutportIsVirtual=on
  ClimaOffReq_ClimaOffReq_write : Outport  Port=136
      settings: EnsureOutportIsVirtual=on
  ClimaPwrCns_ClimaPwrCns_write : Outport  Port=137
      settings: EnsureOutportIsVirtual=on
  ClimaPwrCritDes_ClimaPwrCritDes_write : Outport  Port=138
      settings: EnsureOutportIsVirtual=on
  ClimaPwrNormDes_ClimaPwrNormDes_write : Outport  Port=139
      settings: EnsureOutportIsVirtual=on
  ClimaTiOfEnd_ClimaTiOfEnd_write : Outport  Port=140
      settings: EnsureOutportIsVirtual=on
  CmptModForHp_CmptModForHp_write : Outport  Port=141
      settings: EnsureOutportIsVirtual=on
  CmptmtAirTEstimdAtRowFirstLoResl_CmptmtAirTEstimdAtRowFirstLoResl_write : Outport  Port=142
      settings: EnsureOutportIsVirtual=on
  CmptmtAirTEstimdExtd_CmptmtAirTEstimdExtd_write : Outport  Port=143
      settings: EnsureOutportIsVirtual=on
  CupHoldrActvAllwd_CupHoldrActvAllwd_write : Outport  Port=144
      settings: EnsureOutportIsVirtual=on
  DefrstMaxSts_DefrstMaxSts_write : Outport  Port=145
      settings: EnsureOutportIsVirtual=on
  DrvrSeatHeatgPwrAllwd_DrvrSeatHeatgPwrAllwd_write : Outport  Port=146
      settings: EnsureOutportIsVirtual=on
  DrvrSeatVentnPwrAllwd_DrvrSeatVentnPwrAllwd_write : Outport  Port=147
      settings: EnsureOutportIsVirtual=on
  EcoClimaSts_EcoClimaSts_write : Outport  Port=148
      settings: EnsureOutportIsVirtual=on
  EgyDesForClima_EgyDesForClima_write : Outport  Port=149
      settings: EnsureOutportIsVirtual=on
  ElecDefrstReq_ElecDefrstReq_write : Outport  Port=150
      settings: EnsureOutportIsVirtual=on
  EngRunngReqByClima_EngRunngReqByClima_write : Outport  Port=151
      settings: EnsureOutportIsVirtual=on
  EngSpdIncIdleForClima_EngSpdIncIdleForClima_write : Outport  Port=152
      settings: EnsureOutportIsVirtual=on
  EvaprTFrnt_EvaprTFrnt_write : Outport  Port=153
      settings: EnsureOutportIsVirtual=on
  ExtVentToastFrmClima_ExtVentToastFrmClima_write : Outport  Port=154
      settings: EnsureOutportIsVirtual=on